simulink model "sdfm_filter_sync_cpuread_am263x"  (R2024b)
settings: Solver=FixedStepAuto  StartTime=0.0  StopTime=10.0  RelTol=1e-3  EnableMultiTasking=on
undefined symbols (not defined in the file): SYSCLOCKOUT
libraries: peripheral_library_blocks_am263x x21, HWI_lib x1
(part 2 of 2)

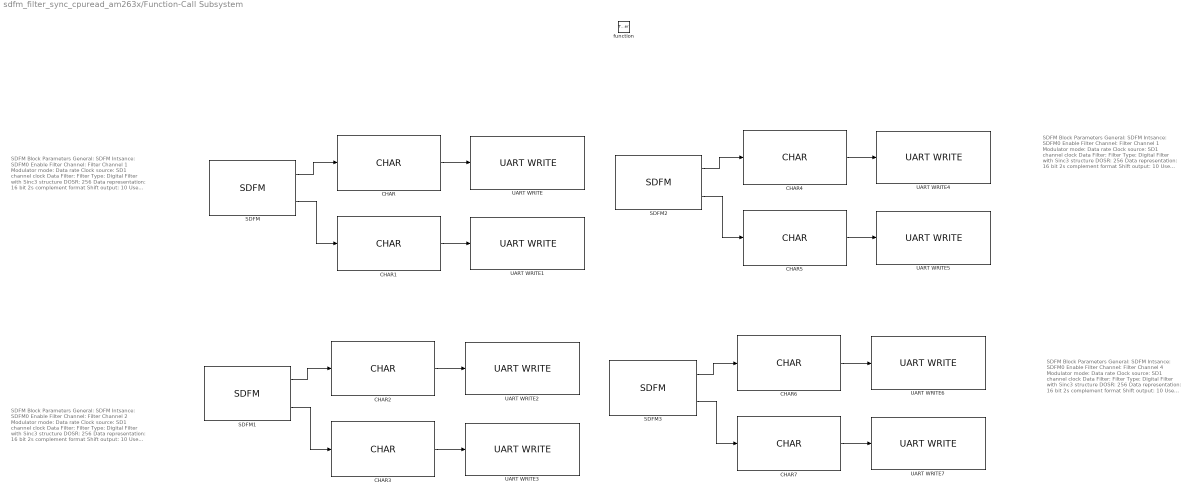
== system sdfm_filter_sync_cpuread_am263x/Function-Call Subsystem ==
  note: SDFM Block Parameters
      |
      | General:
      | SDFM Intsance: SDFM0
      | Enable Filter Channel: Filter Channel 1
      | Modulator mode: Data rate
      | Clock source: SD1 channel clock
      |
      | Data Filter:
      | Filter Type: Digital Filter with Sinc3 structure
      | DOSR: 256
      | Data representation: 16 bit 2s complement format
      | Shift output: 10
      | Use PWM synchronization: True
      | SDSYNC source: PWM1 SOCA
      |
      | Comparator:
      | Filter Type: Digital Filter with Sinc3 structure
      | COSR: 32
      | High level threshold1: 32767
      | High level threshold2: 32767
      | Low level threshold: 0
      | Low level threshold2: 0
      |
      | Interrupt:
      | Enable Filter1 Modulator clock failure interrupt
      | Enable Filter1 SDFM Data Ready Interrupt
  note: SDFM Block Parameters
      |
      | General:
      | SDFM Intsance: SDFM0
      | Enable Filter Channel: Filter Channel 2
      | Modulator mode: Data rate
      | Clock source: SD1 channel clock
      |
      | Data Filter:
      | Filter Type: Digital Filter with Sinc3 structure
      | DOSR: 256
      | Data representation: 16 bit 2s complement format
      | Shift output: 10
      | Use PWM synchronization: True
      | SDSYNC source: PWM1 SOCA
      |
      | Comparator:
      | Filter Type: Digital Filter with Sinc3 structure
      | COSR: 32
      | High level threshold1: 32767
      | High level threshold2: 32767
      | Low level threshold: 0
      | Low level threshold2: 0
      |
      | Interrupt:
      | Enable Filter2 Modulator clock failure interrupt
      | Enable Filter2 SDFM Data Ready Interrupt
  note: SDFM Block Parameters
      |
      | General:
      | SDFM Intsance: SDFM0
      | Enable Filter Channel: Filter Channel 4
      | Modulator mode: Data rate
      | Clock source: SD1 channel clock
      |
      | Data Filter:
      | Filter Type: Digital Filter with Sinc3 structure
      | DOSR: 256
      | Data representation: 16 bit 2s complement format
      | Shift output: 10
      | Use PWM synchronization: True
      | SDSYNC source: PWM1 SOCA
      |
      | Comparator:
      | Filter Type: Digital Filter with Sinc3 structure
      | COSR: 32
      | High level threshold1: 32767
      | High level threshold2: 32767
      | Low level threshold: 0
      | Low level threshold2: 0
      |
      | Interrupt:
      | Enable Filter 4 Modulator clock failure interrupt
      | Enable Filter4 SDFM Data Ready Interrupt
  note: SDFM Block Parameters
      |
      | General:
      | SDFM Intsance: SDFM0
      | Enable Filter Channel: Filter Channel 1
      | Modulator mode: Data rate
      | Clock source: SD1 channel clock
      |
      | Data Filter:
      | Filter Type: Digital Filter with Sinc3 structure
      | DOSR: 256
      | Data representation: 16 bit 2s complement format
      | Shift output: 10
      | Use PWM synchronization: True
      | SDSYNC source: PWM1 SOCA
      |
      | Comparator:
      | Filter Type: Digital Filter with Sinc3 structure
      | COSR: 32
      | High level threshold1: 32767
      | High level threshold2: 32767
      | Low level threshold: 0
      | Low level threshold2: 0
      |
      | Interrupt:
      | Enable Filter3 Modulator clock failure interrupt
      | Enable Filter3 SDFM Data Ready Interrupt
  function : TriggerPort  TriggerType=function-call  VariantControl=(inherit)
  CHAR{,1..7} (8 alike) : peripheral_library_blocks_am263x/CHAR
      settings: SourceProductName=Embedded Coder Support Package for AM263X - Peripheral Blocks
  SDFM : peripheral_library_blocks_am263x/SDFM  SDFM_Instance=SDFM0  Filter_Channel=Filter Channel 1  Filter1_ch1_sdclk_src=SD1 channel clock is the source  Filter1_SD_modulator_mode=Modulator clock is identical to data rate  Filter2_ch2_sdclk_src=Source is respective channel clock  Filter2_SD_modulator_mode=Modulator clock is identical to data rate  Filter3_ch3_sdclk_src=Source is respective channel clock  Filter3_SD_modulator_mode=Modulator clock is identical to data rate  Filter4_ch4_sdclk_src=Source is respective channel clock  Filter4_SD_modulator_mode=Modulator clock is identical to data rate  Comparator_Output=off  SampleTime=-1  Filter1_enable_data_filter=on  Filter1_filter_type=Digital filter with Sinc3 structure  Filter1_DOSR=128  Filter1_data_filter_output_rep=Filter output is in 16 bits 2 complement format  Filter1_Shift=7  Filter1_PWM_Synchronization=off  Filter1_Enable_External_Reset=on  Filter1_Source_SDSYNC_Event=SDFM sync source is PWM1 SOCA  Filter1_clear_wait_sync_flag=off  Filter1_Use_FIFO=off  Filter1_SDFIFO_int_level=FIFO level empty  Filter1_Clear_FIFO_SDSYNC_event=off  Filter2_enable_data_filter=off  Filter2_filter_type=Digital filter with Sinc3 structure  Filter2_DOSR=256  Filter2_data_filter_output_rep=Filter output is in 16 bits 2 complement format  Filter2_Shift=10  Filter2_PWM_Synchronization=off  Filter2_Enable_External_Reset=off  Filter2_Source_SDSYNC_Event=SDFM sync source is PWM0 SOCA  Filter2_clear_wait_sync_flag=off  Filter2_Use_FIFO=off  Filter2_SDFIFO_int_level=FIFO level empty  Filter2_Clear_FIFO_SDSYNC_event=off  Filter3_enable_data_filter=off  Filter3_filter_type=Digital filter with Sinc3 structure  Filter3_DOSR=256  Filter3_data_filter_output_rep=Filter output is in 16 bits 2 complement format  Filter3_Shift=10  Filter3_PWM_Synchronization=off  Filter3_Enable_External_Reset=off  Filter3_Source_SDSYNC_Event=SDFM sync source is PWM0 SOCA  Filter3_clear_wait_sync_flag=off  Filter3_Use_FIFO=off  Filter3_SDFIFO_int_level=FIFO level empty  Filter3_Clear_FIFO_SDSYNC_event=off  Filter4_enable_data_filter=off  Filter4_filter_type=Digital filter with Sinc3 structure  Filter4_DOSR=256  Filter4_data_filter_output_rep=Filter output is in 16 bits 2 complement format  Filter4_Shift=10  Filter4_PWM_Synchronization=off  Filter4_Enable_External_Reset=off  Filter4_Source_SDSYNC_Event=SDFM sync source is PWM0 SOCA  Filter4_clear_wait_sync_flag=off  Filter4_Use_FIFO=off  Filter4_SDFIFO_int_level=FIFO level empty  Filter4_Clear_FIFO_SDSYNC_event=off  Filter1_Enable_Comparator=on  Filter1_CompFilter_Type=Digital filter with Sinc3 structure  Filter1_comp_COSR=32  Filter1_comp_highlvl_threshold1=32767  Filter1_comp_highlvl_threshold2=32767  Filter1_use_high_thresholdz=off  High_level_threshold_z=32767  Filter1_low_level_threshold1=0  Filter1_low_level_threshold2=0  Filter1_Comparator_event1_source_select=COMPH1 event is source  Filter1_use_CEVT1_digital_filter=off  Filter1_Initialize_digital_filter_CEVT1=off  Filter1_CEVT1_prescale=0  Filter1_CEVT1_threshold=0  Filter1_CEVT1_sample_window=0  Filter1_Comparator_event2_source_select=COMPL1 event is source  Filter1_use_CEVT2_digital_filter=off  Filter1_Initialize_digital_filter_CEVT2=off  Filter1_CEVT2_prescale=0  Filter1_CEVT2_threshold=0  Filter1_CEVT2_sample_window=0  Filter2_Enable_Comparator=off  Filter2_CompFilter_Type=Digital filter with Sinc3 structure  Filter2_comp_COSR=32  Filter2_comp_highlvl_threshold1=32767  Filter2_comp_highlvl_threshold2=32767  Filter2_use_high_thresholdz=off  Filter2_High_level_threshold_z=32767  Filter2_low_level_threshold1=0  Filter2_low_level_threshold2=0  Filter2_Comparator_event1_source_select=COMPH1 event is source  Filter2_use_CEVT1_digital_filter=off  Filter2_Initialize_digital_filter_CEVT1=off  Filter2_CEVT1_prescale=0  Filter2_CEVT1_threshold=0  Filter2_CEVT1_sample_window=0  Filter2_Comparator_event2_source_select=COMPL1 event is source  Filter2_use_CEVT2_digital_filter=off  Filter2_Initialize_digital_filter_CEVT2=off  Filter2_CEVT2_prescale=0  Filter2_CEVT2_threshold=0  Filter2_CEVT2_sample_window=0  Filter3_Enable_Comparator=off  Filter3_CompFilter_Type=Digital filter with Sinc3 structure  Filter3_comp_COSR=32  Filter3_comp_highlvl_threshold1=32767  Filter3_comp_highlvl_threshold2=32767  Filter3_use_high_thresholdz=off  Filter3_High_level_threshold_z=32767  Filter3_low_level_threshold1=0  Filter3_low_level_threshold2=0  Filter3_Comparator_event1_source_select=COMPH1 event is source  Filter3_use_CEVT1_digital_filter=off  Filter3_Initialize_digital_filter_CEVT1=off  Filter3_CEVT1_prescale=0  Filter3_CEVT1_threshold=0  Filter3_CEVT1_sample_window=0  Filter3_Comparator_event2_source_select=COMPL1 event is source  Filter3_use_CEVT2_digital_filter=off  Filter3_Initialize_digital_filter_CEVT2=off  Filter3_CEVT2_prescale=0  Filter3_CEVT2_threshold=0  Filter3_CEVT2_sample_window=0  Filter4_Enable_Comparator=off  Filter4_CompFilter_Type=Digital filter with Sinc3 structure  Filter4_comp_COSR=32  Filter4_comp_highlvl_threshold1=32767  Filter4_comp_highlvl_threshold2=32767  Filter4_use_high_thresholdz=off  Filter4_High_level_threshold_z=32767  Filter4_low_level_threshold1=0  Filter4_low_level_threshold2=0  Filter4_Comparator_event1_source_select=COMPH1 event is source  Filter4_use_CEVT1_digital_filter=off  Filter4_Initialize_digital_filter_CEVT1=off  Filter4_CEVT1_prescale=0  Filter4_CEVT1_threshold=0  Filter4_CEVT1_sample_window=0  Filter4_Comparator_event2_source_select=COMPL1 event is source  Filter4_use_CEVT2_digital_filter=off  Filter4_Initialize_digital_filter_CEVT2=off  Filter4_CEVT2_prescale=0  Filter4_CEVT2_threshold=0  Filter4_CEVT2_sample_window=0  Use_SDFM_Interrupts=on  Filter1_ModClock_Interrupt=on  Filter2_ModClock_Interrupt=off  Filter3_ModClock_Interrupt=off  Filter4_ModClock_Interrupt=off  Filter1_SDFM_Data_Ready=on  Filter2_SDFM_Data_Ready=off  Filter3_SDFM_Data_Ready=off  Filter4_SDFM_Data_Ready=off  Filter1_Comparator_Event1_Int=off  Filter2_Comparator_Event1_Int=off  Filter3_Comparator_Event1_Int=off  Filter4_Comparator_Event1_Int=off  Filter1_Comparator_Event2_Int=off  Filter2_Comparator_Event2_Int=off  Filter3_Comparator_Event2_Int=off  Filter4_Comparator_Event2_Int=off  Filter1_FIFO_Overflow_Int=off  Filter2_FIFO_Overflow_Int=off  Filter3_FIFO_Overflow_Int=off  Filter4_FIFO_Overflow_Int=off  Filter1_SDFM_FIFO_Int=off  Filter2_SDFM_FIFO_Int=off  Filter3_SDFM_FIFO_Int=off  Filter4_SDFM_FIFO_Int=off
      settings: SourceProductName=Embedded Coder Support Package for AM263X - Peripheral Blocks
  SDFM1 : peripheral_library_blocks_am263x/SDFM  SDFM_Instance=SDFM0  Filter_Channel=Filter Channel 2  Filter1_ch1_sdclk_src=SD1 channel clock is the source  Filter1_SD_modulator_mode=Modulator clock is identical to data rate  Filter2_ch2_sdclk_src=Source is respective channel clock  Filter2_SD_modulator_mode=Modulator clock is identical to data rate  Filter3_ch3_sdclk_src=Source is respective channel clock  Filter3_SD_modulator_mode=Modulator clock is identical to data rate  Filter4_ch4_sdclk_src=Source is respective channel clock  Filter4_SD_modulator_mode=Modulator clock is identical to data rate  Comparator_Output=off  SampleTime=-1  Filter1_enable_data_filter=on  Filter1_filter_type=Digital filter with Sinc3 structure  Filter1_DOSR=256  Filter1_data_filter_output_rep=Filter output is in 16 bits 2 complement format  Filter1_Shift=10  Filter1_PWM_Synchronization=on  Filter1_Enable_External_Reset=on  Filter1_Source_SDSYNC_Event=SDFM sync source is PWM1 SOCA  Filter1_clear_wait_sync_flag=off  Filter1_Use_FIFO=off  Filter1_SDFIFO_int_level=FIFO level empty  Filter1_Clear_FIFO_SDSYNC_event=off  Filter2_enable_data_filter=on  Filter2_filter_type=Digital filter with Sinc3 structure  Filter2_DOSR=128  Filter2_data_filter_output_rep=Filter output is in 16 bits 2 complement format  Filter2_Shift=7  Filter2_PWM_Synchronization=off  Filter2_Enable_External_Reset=on  Filter2_Source_SDSYNC_Event=SDFM sync source is PWM1 SOCA  Filter2_clear_wait_sync_flag=off  Filter2_Use_FIFO=off  Filter2_SDFIFO_int_level=FIFO level empty  Filter2_Clear_FIFO_SDSYNC_event=off  Filter3_enable_data_filter=off  Filter3_filter_type=Digital filter with Sinc3 structure  Filter3_DOSR=256  Filter3_data_filter_output_rep=Filter output is in 16 bits 2 complement format  Filter3_Shift=10  Filter3_PWM_Synchronization=off  Filter3_Enable_External_Reset=off  Filter3_Source_SDSYNC_Event=SDFM sync source is PWM0 SOCA  Filter3_clear_wait_sync_flag=off  Filter3_Use_FIFO=off  Filter3_SDFIFO_int_level=FIFO level empty  Filter3_Clear_FIFO_SDSYNC_event=off  Filter4_enable_data_filter=off  Filter4_filter_type=Digital filter with Sinc3 structure  Filter4_DOSR=256  Filter4_data_filter_output_rep=Filter output is in 16 bits 2 complement format  Filter4_Shift=10  Filter4_PWM_Synchronization=off  Filter4_Enable_External_Reset=off  Filter4_Source_SDSYNC_Event=SDFM sync source is PWM0 SOCA  Filter4_clear_wait_sync_flag=off  Filter4_Use_FIFO=off  Filter4_SDFIFO_int_level=FIFO level empty  Filter4_Clear_FIFO_SDSYNC_event=off  Filter1_Enable_Comparator=on  Filter1_CompFilter_Type=Digital filter with Sinc3 structure  Filter1_comp_COSR=32  Filter1_comp_highlvl_threshold1=32767  Filter1_comp_highlvl_threshold2=32767  Filter1_use_high_thresholdz=off  High_level_threshold_z=32767  Filter1_low_level_threshold1=0  Filter1_low_level_threshold2=0  Filter1_Comparator_event1_source_select=COMPH1 event is source  Filter1_use_CEVT1_digital_filter=off  Filter1_Initialize_digital_filter_CEVT1=off  Filter1_CEVT1_prescale=0  Filter1_CEVT1_threshold=0  Filter1_CEVT1_sample_window=0  Filter1_Comparator_event2_source_select=COMPL1 event is source  Filter1_use_CEVT2_digital_filter=off  Filter1_Initialize_digital_filter_CEVT2=off  Filter1_CEVT2_prescale=0  Filter1_CEVT2_threshold=0  Filter1_CEVT2_sample_window=0  Filter2_Enable_Comparator=on  Filter2_CompFilter_Type=Digital filter with Sinc3 structure  Filter2_comp_COSR=32  Filter2_comp_highlvl_threshold1=32767  Filter2_comp_highlvl_threshold2=32767  Filter2_use_high_thresholdz=off  Filter2_High_level_threshold_z=32767  Filter2_low_level_threshold1=0  Filter2_low_level_threshold2=0  Filter2_Comparator_event1_source_select=COMPH1 event is source  Filter2_use_CEVT1_digital_filter=off  Filter2_Initialize_digital_filter_CEVT1=off  Filter2_CEVT1_prescale=0  Filter2_CEVT1_threshold=0  Filter2_CEVT1_sample_window=0  Filter2_Comparator_event2_source_select=COMPL1 event is source  Filter2_use_CEVT2_digital_filter=off  Filter2_Initialize_digital_filter_CEVT2=off  Filter2_CEVT2_prescale=0  Filter2_CEVT2_threshold=0  Filter2_CEVT2_sample_window=0  Filter3_Enable_Comparator=off  Filter3_CompFilter_Type=Digital filter with Sinc3 structure  Filter3_comp_COSR=32  Filter3_comp_highlvl_threshold1=32767  Filter3_comp_highlvl_threshold2=32767  Filter3_use_high_thresholdz=off  Filter3_High_level_threshold_z=32767  Filter3_low_level_threshold1=0  Filter3_low_level_threshold2=0  Filter3_Comparator_event1_source_select=COMPH1 event is source  Filter3_use_CEVT1_digital_filter=off  Filter3_Initialize_digital_filter_CEVT1=off  Filter3_CEVT1_prescale=0  Filter3_CEVT1_threshold=0  Filter3_CEVT1_sample_window=0  Filter3_Comparator_event2_source_select=COMPL1 event is source  Filter3_use_CEVT2_digital_filter=off  Filter3_Initialize_digital_filter_CEVT2=off  Filter3_CEVT2_prescale=0  Filter3_CEVT2_threshold=0  Filter3_CEVT2_sample_window=0  Filter4_Enable_Comparator=off  Filter4_CompFilter_Type=Digital filter with Sinc3 structure  Filter4_comp_COSR=32  Filter4_comp_highlvl_threshold1=32767  Filter4_comp_highlvl_threshold2=32767  Filter4_use_high_thresholdz=off  Filter4_High_level_threshold_z=32767  Filter4_low_level_threshold1=0  Filter4_low_level_threshold2=0  Filter4_Comparator_event1_source_select=COMPH1 event is source  Filter4_use_CEVT1_digital_filter=off  Filter4_Initialize_digital_filter_CEVT1=off  Filter4_CEVT1_prescale=0  Filter4_CEVT1_threshold=0  Filter4_CEVT1_sample_window=0  Filter4_Comparator_event2_source_select=COMPL1 event is source  Filter4_use_CEVT2_digital_filter=off  Filter4_Initialize_digital_filter_CEVT2=off  Filter4_CEVT2_prescale=0  Filter4_CEVT2_threshold=0  Filter4_CEVT2_sample_window=0  Use_SDFM_Interrupts=on  Filter1_ModClock_Interrupt=on  Filter2_ModClock_Interrupt=on  Filter3_ModClock_Interrupt=off  Filter4_ModClock_Interrupt=off  Filter1_SDFM_Data_Ready=on  Filter2_SDFM_Data_Ready=on  Filter3_SDFM_Data_Ready=off  Filter4_SDFM_Data_Ready=off  Filter1_Comparator_Event1_Int=off  Filter2_Comparator_Event1_Int=off  Filter3_Comparator_Event1_Int=off  Filter4_Comparator_Event1_Int=off  Filter1_Comparator_Event2_Int=off  Filter2_Comparator_Event2_Int=off  Filter3_Comparator_Event2_Int=off  Filter4_Comparator_Event2_Int=off  Filter1_FIFO_Overflow_Int=off  Filter2_FIFO_Overflow_Int=off  Filter3_FIFO_Overflow_Int=off  Filter4_FIFO_Overflow_Int=off  Filter1_SDFM_FIFO_Int=off  Filter2_SDFM_FIFO_Int=off  Filter3_SDFM_FIFO_Int=off  Filter4_SDFM_FIFO_Int=off
      settings: SourceProductName=Embedded Coder Support Package for AM263X - Peripheral Blocks
  SDFM2 : peripheral_library_blocks_am263x/SDFM  SDFM_Instance=SDFM0  Filter_Channel=Filter Channel 3  Filter1_ch1_sdclk_src=SD1 channel clock is the source  Filter1_SD_modulator_mode=Modulator clock is identical to data rate  Filter2_ch2_sdclk_src=Source is respective channel clock  Filter2_SD_modulator_mode=Modulator clock is identical to data rate  Filter3_ch3_sdclk_src=Source is respective channel clock  Filter3_SD_modulator_mode=Modulator clock is identical to data rate  Filter4_ch4_sdclk_src=Source is respective channel clock  Filter4_SD_modulator_mode=Modulator clock is identical to data rate  Comparator_Output=off  SampleTime=-1  Filter1_enable_data_filter=on  Filter1_filter_type=Digital filter with Sinc3 structure  Filter1_DOSR=256  Filter1_data_filter_output_rep=Filter output is in 16 bits 2 complement format  Filter1_Shift=10  Filter1_PWM_Synchronization=on  Filter1_Enable_External_Reset=on  Filter1_Source_SDSYNC_Event=SDFM sync source is PWM1 SOCA  Filter1_clear_wait_sync_flag=off  Filter1_Use_FIFO=off  Filter1_SDFIFO_int_level=FIFO level empty  Filter1_Clear_FIFO_SDSYNC_event=off  Filter2_enable_data_filter=off  Filter2_filter_type=Digital filter with Sinc3 structure  Filter2_DOSR=256  Filter2_data_filter_output_rep=Filter output is in 16 bits 2 complement format  Filter2_Shift=10  Filter2_PWM_Synchronization=off  Filter2_Enable_External_Reset=off  Filter2_Source_SDSYNC_Event=SDFM sync source is PWM0 SOCA  Filter2_clear_wait_sync_flag=off  Filter2_Use_FIFO=off  Filter2_SDFIFO_int_level=FIFO level empty  Filter2_Clear_FIFO_SDSYNC_event=off  Filter3_enable_data_filter=on  Filter3_filter_type=Digital filter with Sinc3 structure  Filter3_DOSR=128  Filter3_data_filter_output_rep=Filter output is in 16 bits 2 complement format  Filter3_Shift=7  Filter3_PWM_Synchronization=off  Filter3_Enable_External_Reset=on  Filter3_Source_SDSYNC_Event=SDFM sync source is PWM1 SOCA  Filter3_clear_wait_sync_flag=off  Filter3_Use_FIFO=off  Filter3_SDFIFO_int_level=FIFO level empty  Filter3_Clear_FIFO_SDSYNC_event=off  Filter4_enable_data_filter=off  Filter4_filter_type=Digital filter with Sinc3 structure  Filter4_DOSR=256  Filter4_data_filter_output_rep=Filter output is in 16 bits 2 complement format  Filter4_Shift=10  Filter4_PWM_Synchronization=off  Filter4_Enable_External_Reset=off  Filter4_Source_SDSYNC_Event=SDFM sync source is PWM0 SOCA  Filter4_clear_wait_sync_flag=off  Filter4_Use_FIFO=off  Filter4_SDFIFO_int_level=FIFO level empty  Filter4_Clear_FIFO_SDSYNC_event=off  Filter1_Enable_Comparator=on  Filter1_CompFilter_Type=Digital filter with Sinc3 structure  Filter1_comp_COSR=32  Filter1_comp_highlvl_threshold1=32767  Filter1_comp_highlvl_threshold2=32767  Filter1_use_high_thresholdz=off  High_level_threshold_z=32767  Filter1_low_level_threshold1=0  Filter1_low_level_threshold2=0  Filter1_Comparator_event1_source_select=COMPH1 event is source  Filter1_use_CEVT1_digital_filter=off  Filter1_Initialize_digital_filter_CEVT1=off  Filter1_CEVT1_prescale=0  Filter1_CEVT1_threshold=0  Filter1_CEVT1_sample_window=0  Filter1_Comparator_event2_source_select=COMPL1 event is source  Filter1_use_CEVT2_digital_filter=off  Filter1_Initialize_digital_filter_CEVT2=off  Filter1_CEVT2_prescale=0  Filter1_CEVT2_threshold=0  Filter1_CEVT2_sample_window=0  Filter2_Enable_Comparator=off  Filter2_CompFilter_Type=Digital filter with Sinc3 structure  Filter2_comp_COSR=32  Filter2_comp_highlvl_threshold1=32767  Filter2_comp_highlvl_threshold2=32767  Filter2_use_high_thresholdz=off  Filter2_High_level_threshold_z=32767  Filter2_low_level_threshold1=0  Filter2_low_level_threshold2=0  Filter2_Comparator_event1_source_select=COMPH1 event is source  Filter2_use_CEVT1_digital_filter=off  Filter2_Initialize_digital_filter_CEVT1=off  Filter2_CEVT1_prescale=0  Filter2_CEVT1_threshold=0  Filter2_CEVT1_sample_window=0  Filter2_Comparator_event2_source_select=COMPL1 event is source  Filter2_use_CEVT2_digital_filter=off  Filter2_Initialize_digital_filter_CEVT2=off  Filter2_CEVT2_prescale=0  Filter2_CEVT2_threshold=0  Filter2_CEVT2_sample_window=0  Filter3_Enable_Comparator=on  Filter3_CompFilter_Type=Digital filter with Sinc3 structure  Filter3_comp_COSR=32  Filter3_comp_highlvl_threshold1=32767  Filter3_comp_highlvl_threshold2=32767  Filter3_use_high_thresholdz=off  Filter3_High_level_threshold_z=32767  Filter3_low_level_threshold1=0  Filter3_low_level_threshold2=0  Filter3_Comparator_event1_source_select=COMPH1 event is source  Filter3_use_CEVT1_digital_filter=off  Filter3_Initialize_digital_filter_CEVT1=off  Filter3_CEVT1_prescale=0  Filter3_CEVT1_threshold=0  Filter3_CEVT1_sample_window=0  Filter3_Comparator_event2_source_select=COMPL1 event is source  Filter3_use_CEVT2_digital_filter=off  Filter3_Initialize_digital_filter_CEVT2=off  Filter3_CEVT2_prescale=0  Filter3_CEVT2_threshold=0  Filter3_CEVT2_sample_window=0  Filter4_Enable_Comparator=off  Filter4_CompFilter_Type=Digital filter with Sinc3 structure  Filter4_comp_COSR=32  Filter4_comp_highlvl_threshold1=32767  Filter4_comp_highlvl_threshold2=32767  Filter4_use_high_thresholdz=off  Filter4_High_level_threshold_z=32767  Filter4_low_level_threshold1=0  Filter4_low_level_threshold2=0  Filter4_Comparator_event1_source_select=COMPH1 event is source  Filter4_use_CEVT1_digital_filter=off  Filter4_Initialize_digital_filter_CEVT1=off  Filter4_CEVT1_prescale=0  Filter4_CEVT1_threshold=0  Filter4_CEVT1_sample_window=0  Filter4_Comparator_event2_source_select=COMPL1 event is source  Filter4_use_CEVT2_digital_filter=off  Filter4_Initialize_digital_filter_CEVT2=off  Filter4_CEVT2_prescale=0  Filter4_CEVT2_threshold=0  Filter4_CEVT2_sample_window=0  Use_SDFM_Interrupts=on  Filter1_ModClock_Interrupt=on  Filter2_ModClock_Interrupt=off  Filter3_ModClock_Interrupt=on  Filter4_ModClock_Interrupt=off  Filter1_SDFM_Data_Ready=on  Filter2_SDFM_Data_Ready=off  Filter3_SDFM_Data_Ready=on  Filter4_SDFM_Data_Ready=off  Filter1_Comparator_Event1_Int=off  Filter2_Comparator_Event1_Int=off  Filter3_Comparator_Event1_Int=off  Filter4_Comparator_Event1_Int=off  Filter1_Comparator_Event2_Int=off  Filter2_Comparator_Event2_Int=off  Filter3_Comparator_Event2_Int=off  Filter4_Comparator_Event2_Int=off  Filter1_FIFO_Overflow_Int=off  Filter2_FIFO_Overflow_Int=off  Filter3_FIFO_Overflow_Int=off  Filter4_FIFO_Overflow_Int=off  Filter1_SDFM_FIFO_Int=off  Filter2_SDFM_FIFO_Int=off  Filter3_SDFM_FIFO_Int=off  Filter4_SDFM_FIFO_Int=off
      settings: SourceProductName=Embedded Coder Support Package for AM263X - Peripheral Blocks
  SDFM3 : peripheral_library_blocks_am263x/SDFM  SDFM_Instance=SDFM0  Filter_Channel=Filter Channel 4  Filter1_ch1_sdclk_src=SD1 channel clock is the source  Filter1_SD_modulator_mode=Modulator clock is identical to data rate  Filter2_ch2_sdclk_src=SD1 channel clock is the source  Filter2_SD_modulator_mode=Modulator clock is identical to data rate  Filter3_ch3_sdclk_src=Source is respective channel clock  Filter3_SD_modulator_mode=Modulator clock is identical to data rate  Filter4_ch4_sdclk_src=Source is respective channel clock  Filter4_SD_modulator_mode=Modulator clock is identical to data rate  Comparator_Output=off  SampleTime=-1  Filter1_enable_data_filter=on  Filter1_filter_type=Digital filter with Sinc3 structure  Filter1_DOSR=256  Filter1_data_filter_output_rep=Filter output is in 16 bits 2 complement format  Filter1_Shift=10  Filter1_PWM_Synchronization=on  Filter1_Enable_External_Reset=on  Filter1_Source_SDSYNC_Event=SDFM sync source is PWM1 SOCA  Filter1_clear_wait_sync_flag=off  Filter1_Use_FIFO=off  Filter1_SDFIFO_int_level=FIFO level empty  Filter1_Clear_FIFO_SDSYNC_event=off  Filter2_enable_data_filter=on  Filter2_filter_type=Digital filter with Sinc3 structure  Filter2_DOSR=256  Filter2_data_filter_output_rep=Filter output is in 16 bits 2 complement format  Filter2_Shift=10  Filter2_PWM_Synchronization=on  Filter2_Enable_External_Reset=on  Filter2_Source_SDSYNC_Event=SDFM sync source is PWM1 SOCA  Filter2_clear_wait_sync_flag=off  Filter2_Use_FIFO=off  Filter2_SDFIFO_int_level=FIFO level empty  Filter2_Clear_FIFO_SDSYNC_event=off  Filter3_enable_data_filter=off  Filter3_filter_type=Digital filter with Sinc3 structure  Filter3_DOSR=256  Filter3_data_filter_output_rep=Filter output is in 16 bits 2 complement format  Filter3_Shift=10  Filter3_PWM_Synchronization=off  Filter3_Enable_External_Reset=off  Filter3_Source_SDSYNC_Event=SDFM sync source is PWM0 SOCA  Filter3_clear_wait_sync_flag=off  Filter3_Use_FIFO=off  Filter3_SDFIFO_int_level=FIFO level empty  Filter3_Clear_FIFO_SDSYNC_event=off  Filter4_enable_data_filter=on  Filter4_filter_type=Digital filter with Sinc3 structure  Filter4_DOSR=128  Filter4_data_filter_output_rep=Filter output is in 16 bits 2 complement format  Filter4_Shift=7  Filter4_PWM_Synchronization=off  Filter4_Enable_External_Reset=on  Filter4_Source_SDSYNC_Event=SDFM sync source is PWM0 SOCA  Filter4_clear_wait_sync_flag=off  Filter4_Use_FIFO=off  Filter4_SDFIFO_int_level=FIFO level empty  Filter4_Clear_FIFO_SDSYNC_event=off  Filter1_Enable_Comparator=on  Filter1_CompFilter_Type=Digital filter with Sinc3 structure  Filter1_comp_COSR=32  Filter1_comp_highlvl_threshold1=32767  Filter1_comp_highlvl_threshold2=32767  Filter1_use_high_thresholdz=off  High_level_threshold_z=32767  Filter1_low_level_threshold1=0  Filter1_low_level_threshold2=0  Filter1_Comparator_event1_source_select=COMPH1 event is source  Filter1_use_CEVT1_digital_filter=off  Filter1_Initialize_digital_filter_CEVT1=off  Filter1_CEVT1_prescale=0  Filter1_CEVT1_threshold=0  Filter1_CEVT1_sample_window=0  Filter1_Comparator_event2_source_select=COMPL1 event is source  Filter1_use_CEVT2_digital_filter=off  Filter1_Initialize_digital_filter_CEVT2=off  Filter1_CEVT2_prescale=0  Filter1_CEVT2_threshold=0  Filter1_CEVT2_sample_window=0  Filter2_Enable_Comparator=on  Filter2_CompFilter_Type=Digital filter with Sinc3 structure  Filter2_comp_COSR=32  Filter2_comp_highlvl_threshold1=32767  Filter2_comp_highlvl_threshold2=32767  Filter2_use_high_thresholdz=off  Filter2_High_level_threshold_z=32767  Filter2_low_level_threshold1=0  Filter2_low_level_threshold2=0  Filter2_Comparator_event1_source_select=COMPH1 event is source  Filter2_use_CEVT1_digital_filter=off  Filter2_Initialize_digital_filter_CEVT1=off  Filter2_CEVT1_prescale=0  Filter2_CEVT1_threshold=0  Filter2_CEVT1_sample_window=0  Filter2_Comparator_event2_source_select=COMPL1 event is source  Filter2_use_CEVT2_digital_filter=off  Filter2_Initialize_digital_filter_CEVT2=off  Filter2_CEVT2_prescale=0  Filter2_CEVT2_threshold=0  Filter2_CEVT2_sample_window=0  Filter3_Enable_Comparator=off  Filter3_CompFilter_Type=Digital filter with Sinc3 structure  Filter3_comp_COSR=32  Filter3_comp_highlvl_threshold1=32767  Filter3_comp_highlvl_threshold2=32767  Filter3_use_high_thresholdz=off  Filter3_High_level_threshold_z=32767  Filter3_low_level_threshold1=0  Filter3_low_level_threshold2=0  Filter3_Comparator_event1_source_select=COMPH1 event is source  Filter3_use_CEVT1_digital_filter=off  Filter3_Initialize_digital_filter_CEVT1=off  Filter3_CEVT1_prescale=0  Filter3_CEVT1_threshold=0  Filter3_CEVT1_sample_window=0  Filter3_Comparator_event2_source_select=COMPL1 event is source  Filter3_use_CEVT2_digital_filter=off  Filter3_Initialize_digital_filter_CEVT2=off  Filter3_CEVT2_prescale=0  Filter3_CEVT2_threshold=0  Filter3_CEVT2_sample_window=0  Filter4_Enable_Comparator=on  Filter4_CompFilter_Type=Digital filter with Sinc3 structure  Filter4_comp_COSR=32  Filter4_comp_highlvl_threshold1=32767  Filter4_comp_highlvl_threshold2=32767  Filter4_use_high_thresholdz=off  Filter4_High_level_threshold_z=32767  Filter4_low_level_threshold1=0  Filter4_low_level_threshold2=0  Filter4_Comparator_event1_source_select=COMPH1 event is source  Filter4_use_CEVT1_digital_filter=off  Filter4_Initialize_digital_filter_CEVT1=off  Filter4_CEVT1_prescale=0  Filter4_CEVT1_threshold=0  Filter4_CEVT1_sample_window=0  Filter4_Comparator_event2_source_select=COMPL1 event is source  Filter4_use_CEVT2_digital_filter=off  Filter4_Initialize_digital_filter_CEVT2=off  Filter4_CEVT2_prescale=0  Filter4_CEVT2_threshold=0  Filter4_CEVT2_sample_window=0  Use_SDFM_Interrupts=on  Filter1_ModClock_Interrupt=on  Filter2_ModClock_Interrupt=on  Filter3_ModClock_Interrupt=off  Filter4_ModClock_Interrupt=on  Filter1_SDFM_Data_Ready=on  Filter2_SDFM_Data_Ready=on  Filter3_SDFM_Data_Ready=off  Filter4_SDFM_Data_Ready=on  Filter1_Comparator_Event1_Int=off  Filter2_Comparator_Event1_Int=off  Filter3_Comparator_Event1_Int=off  Filter4_Comparator_Event1_Int=off  Filter1_Comparator_Event2_Int=off  Filter2_Comparator_Event2_Int=off  Filter3_Comparator_Event2_Int=off  Filter4_Comparator_Event2_Int=off  Filter1_FIFO_Overflow_Int=off  Filter2_FIFO_Overflow_Int=off  Filter3_FIFO_Overflow_Int=off  Filter4_FIFO_Overflow_Int=off  Filter1_SDFM_FIFO_Int=off  Filter2_SDFM_FIFO_Int=off  Filter3_SDFM_FIFO_Int=off  Filter4_SDFM_FIFO_Int=off
      settings: SourceProductName=Embedded Coder Support Package for AM263X - Peripheral Blocks
  UART WRITE{,1..7} (8 alike) : peripheral_library_blocks_am263x/UART WRITE  UARTPort=UART0
      settings: SourceProductName=Embedded Coder Support Package for AM263X - Peripheral Blocks
  wires:
  SDFM/1 -> CHAR/1
  SDFM/2 -> CHAR1/1
  CHAR/1 -> UART WRITE/1
  CHAR1/1 -> UART WRITE1/1
  SDFM1/1 -> CHAR2/1
  CHAR2/1 -> UART WRITE2/1
  CHAR3/1 -> UART WRITE3/1
  SDFM1/2 -> CHAR3/1
  SDFM2/1 -> CHAR4/1
  CHAR4/1 -> UART WRITE4/1
  CHAR5/1 -> UART WRITE5/1
  SDFM2/2 -> CHAR5/1
  CHAR7/1 -> UART WRITE7/1
  CHAR6/1 -> UART WRITE6/1
  SDFM3/2 -> CHAR7/1
  SDFM3/1 -> CHAR6/1
